simulink library "inp"  (Simulink 8.2)
settings: Solver=ode45  SolverMode=SingleTasking  StartTime=0.0  StopTime=10.0  RelTol=1e-3
undefined symbols (not defined in the file or in its folder): C_TICK_TIME_S, ENUM_FAULTSTATE_FAIL, ENUM_FAULTSTATE_INDETERMINATE, ENUM_FAULTSTATE_PASS, ENUM_KEYSWITCH_ON, FALSE, KINP_ABS1CANCommuOk_flg_ovrdflg, KINP_ABS1CANCommuOk_flg_ovrdval, KINP_ABS1CANPeriodTime_s, KINP_ABS1CANRolCntOk_flg_ovrdflg, KINP_ABS1CANRolCntOk_flg_ovrdval, KINP_ABS2CANChkSumOk_flg_ovrdflg, KINP_ABS2CANChkSumOk_flg_ovrdval, KINP_ABS2CANCommuOk_flg_ovrdflg, KINP_ABS2CANCommuOk_flg_ovrdval, KINP_ABS2CANPeriodTime_s, KINP_ABS2CANRolCntOk_flg_ovrdflg, KINP_ABS2CANRolCntOk_flg_ovrdval, KINP_ABS3CANCommuOk_flg_ovrdflg, KINP_ABS3CANCommuOk_flg_ovrdval, KINP_ABS4CANCommuOk_flg_ovrdflg, KINP_ABS4CANCommuOk_flg_ovrdval, KINP_ABSActive_flg_defval, KINP_ABSActive_flg_invalid, KINP_ABSActive_flg_ovrdflg, KINP_ABSActive_flg_ovrdval, KINP_ABSActive_flg_setvalid, KINP_ABSActive_flg_srvcflg, KINP_ABSActive_flg_srvcval, KINP_ABSCANAlive_flg_ovrdflg, KINP_ABSCANAlive_flg_ovrdval, KINP_ABSCANCommuOk_flg_ovrdflg, KINP_ABSCANCommuOk_flg_ovrdval, KINP_ABSCANValidOk_flg_ovrdflg, KINP_ABSCANValidOk_flg_ovrdval, KINP_ABSFault_flg_defval, KINP_ABSFault_flg_invalid, KINP_ABSFault_flg_ovrdflg, KINP_ABSFault_flg_ovrdval, KINP_ABSFault_flg_setvalid, KINP_ABSFault_flg_srvcflg, KINP_ABSFault_flg_srvcval, KINP_AC1CANCommuOk_flg_ovrdflg, KINP_AC1CANCommuOk_flg_ovrdval, KINP_ACCANAlive_flg_ovrdflg, KINP_ACCANAlive_flg_ovrdval, KINP_ACCANCommuOk_flg_ovrdflg, KINP_ACCANCommuOk_flg_ovrdval, KINP_ACCANValidOk_flg_ovrdflg, KINP_ACCANValidOk_flg_ovrdval, KINP_ACCCancelH_flg_defval, KINP_ACCCancelH_flg_invalid, KINP_ACCCancelH_flg_ovrdflg, KINP_ACCCancelH_flg_ovrdval, KINP_ACCCancelH_flg_setvalid, KINP_ACCCancelH_flg_srvcflg, KINP_ACCCancelH_flg_srvcval, KINP_AccelPedalPosn_pct_defval, KINP_AccelPedalPosn_pct_gain, KINP_AccelPedalPosn_pct_invalid ... (+1866)
libraries: inp x192, hcu_lib x34, simulink x33
(part 6 of 21)

== system inp/hw_signal_process/sig_valid_check ==
  input_signal_filt : Inport
  adc_fault : Inport  Port=2
  vref_fault : Inport  Port=3
  range_high_ifault_flg : Inport  Port=4
  range_low_ifault_flg : Inport  Port=5
  lim : Inport  Port=6
  inc : Inport  Port=7
  dec : Inport  Port=8
  def_value : Inport  Port=9
  Logical Operator1 : Logic  Operator=OR  Inputs=4  AllPortsSameDT=off  OutDataTypeStr=boolean
  Logical Operator2 : Logic  Operator=OR  Inputs=4  AllPortsSameDT=off  OutDataTypeStr=boolean
  Logical Operator3 : Logic  Operator=OR  AllPortsSameDT=off  OutDataTypeStr=boolean
  Relational Operator{,1..3} (4 alike) : RelationalOperator  Operator===  OutDataTypeStr=boolean
  Switch{,1..5} (6 alike) : Switch  Criteria=u2 ~= 0
  Terminator{1..4} (4 alike) : Terminator
  Unit Delay : UnitDelay  SampleTime=-1
  resetting_fault_filter{,1..3} (4 alike) : hcu_lib/fault_filter_reset
  zero{,1,4,8} (4 alike) : Constant  Tag=struct('comment',struct('text','','placement',1),'output',struct('width',1,'name','$S_$B','unit','','type','Int16','offset',0,'max',NaN,'min',NaN,'freeze',1,'lsb',1,'arb',0,'class','default','address','','description','','value','0','tolerance',1,'show',0),'date',731455.58675544)  Value=ENUM_FAULTSTATE_FAIL
  zero{2..3,6..7} (4 alike) : Constant  Tag=struct('comment',struct('text','','placement',1),'output',struct('width',1,'name','$S_$B','unit','','type','Int16','offset',0,'max',NaN,'min',NaN,'freeze',1,'lsb',1,'arb',0,'class','default','address','','description','','value','0','tolerance',1,'show',0),'date',731455.58675544)  Value=ENUM_FAULTSTATE_INDETERMINATE
  zero5 : Constant  Tag=struct('comment',struct('text','','placement',1),'output',struct('width',1,'name','$S_$B','unit','','type','Int16','offset',0,'max',NaN,'min',NaN,'freeze',1,'lsb',1,'arb',0,'class','default','address','','description','','value','0','tolerance',1,'show',0),'date',731455.58675544)  Value=ENUM_FAULTSTATE_PASS
  zero9 : Constant  Tag=struct('comment',struct('text','','placement',1),'output',struct('width',1,'name','$S_$B','unit','','type','Int16','offset',0,'max',NaN,'min',NaN,'freeze',1,'lsb',1,'arb',0,'class','default','address','','description','','value','0','tolerance',1,'show',0),'date',731455.58675544)  Value=ENUM_FAULTSTATE_PASS
  range_high_flt : Outport
  range_low_flt : Outport  Port=2
  fault_flg : Outport  Port=3
  valid_signal : Outport  Port=4
  wires:
  Unit Delay/1 -> Switch1/1
  [fault_flg] -> Switch/2
  [inst_low_fault] -> resetting_fault_filter2/1
  Switch/1 -> Unit Delay/1, valid_signal/1
  Switch1/1 -> Switch/3
  def_value/1 -> Switch/1
  dec/1 -> resetting_fault_filter/4, [dec]
  inc/1 -> resetting_fault_filter/3, [inc]
  lim/1 -> resetting_fault_filter/2, [lim]
  range_high_ifault_flg/1 -> [inst_high_fault]
  input_signal_filt/1 -> Switch1/3
  Logical Operator1/1 -> Switch1/2  "ifault_flg"
  range_low_ifault_flg/1 -> [inst_low_fault]
  resetting_fault_filter2/1 -> [low_fault_flg]
  resetting_fault_filter/2 -> Terminator1/1
  resetting_fault_filter2/2 -> Terminator2/1
  [lim] -> resetting_fault_filter2/2
  [inc] -> resetting_fault_filter2/3
  [dec] -> resetting_fault_filter2/4
  adc_fault/1 -> Relational Operator/1, [adc_fault]
  zero/1 -> Relational Operator/2
  zero1/1 -> Relational Operator1/2
  vref_fault/1 -> Relational Operator1/1, [vref_fault]
  Relational Operator/1 -> [inst_adc_fault]
  Relational Operator1/1 -> [inst_vref_fault]
  [lim] -> resetting_fault_filter1/2
  [inc] -> resetting_fault_filter1/3
  [dec] -> resetting_fault_filter1/4
  [inst_vref_fault] -> resetting_fault_filter3/1
  [lim] -> resetting_fault_filter3/2
  [inc] -> resetting_fault_filter3/3
  [dec] -> resetting_fault_filter3/4
  resetting_fault_filter1/2 -> Terminator3/1
  resetting_fault_filter3/2 -> Terminator4/1
  resetting_fault_filter3/1 -> [vref_fault_flg]
  [inst_adc_fault] -> resetting_fault_filter/1
  resetting_fault_filter/1 -> [adc_fault_flg]
  [inst_high_fault] -> resetting_fault_filter1/1
  resetting_fault_filter1/1 -> [high_fault_flg]
  [adc_fault_flg] -> Logical Operator2/1
  [vref_fault_flg] -> Logical Operator2/2
  [high_fault_flg] -> Logical Operator2/3
  [low_fault_flg] -> Logical Operator2/4
  Logical Operator2/1 -> fault_flg/1, [fault_flg]  "fault_flg"
  [inst_adc_fault] -> Logical Operator1/1
  [inst_vref_fault] -> Logical Operator1/2
  [inst_high_fault] -> Logical Operator1/3
  [inst_low_fault] -> Logical Operator1/4
  zero2/1 -> Relational Operator2/2
  [high_fault_flg] -> Switch3/2
  Switch3/1 -> Switch2/3
  zero3/1 -> Switch2/1
  [adc_fault] -> Relational Operator2/1
  zero4/1 -> Switch3/1
  zero5/1 -> Switch3/3
  Switch2/1 -> range_high_flt/1
  zero6/1 -> Relational Operator3/2
  [vref_fault] -> Relational Operator3/1
  Relational Operator2/1 -> Logical Operator3/1
  Relational Operator3/1 -> Logical Operator3/2
  [indeterminate] -> Switch2/2
  Logical Operator3/1 -> [indeterminate]
  [low_fault_flg] -> Switch5/2
  Switch5/1 -> Switch4/3
  zero7/1 -> Switch4/1
  zero8/1 -> Switch5/1
  zero9/1 -> Switch5/3
  [indeterminate] -> Switch4/2
  Switch4/1 -> range_low_flt/1

== system inp/hw_signal_process/signal_filter ==
  raw_input : Inport
  Tc : Inport  Port=2
  Ts : Inport  Port=3
  FirstOrderFilter : hcu_lib/FirstOrderFilter  SystemSampleTime=-1
  IC : UnitDelay  Tag=struct('comment',struct('text','','placement',1),'logdata',struct('maxnumvals',inf,'plotchannels',[],'stepsize',0,'downsampling',1,'loggingmode',3,'channels',1),'output',struct('width',1,'name','$S_$B','unit','','type','Int16','offset',0,'max',NaN,'min',NaN,'freeze',1,'lsb',1,'arb',0,'class','default','address','','description','','autoscalingmode',1,'lowerheadroom',1,'upperheadroom',1,'headroomunit','bit','checkmin',0,'checkmax',0,'show',0,'initial','2'),'state',0,'dt','-1','date',731455.586925799)  SampleTime=-1
  Switch1 : Switch  Tag=struct('comment',struct('text','','placement',1),'logdata',struct('maxnumvals',inf,'plotchannels',[],'stepsize',0,'downsampling',1,'loggingmode',3,'channels',1),'output',struct('width',1,'name','$S_$B','unit','','type','Int32Bin15','offset',0,'max',65535,'min',-65536,'freeze',1,'lsb',2^-15,'arb',0,'class','default','address','','description','','autoscalingmode',1,'lowerheadroom',1,'upperheadroom',1,'headroomunit','bit','checkmin',0,'checkmax',0,'show',0),'threshold',struct('width',1,'name','$S_$B_threshold','unit','','type','Int16','offset',0,'max',NaN,'min',NaN,'freeze',1,'lsb',1,'arb',0,'class','default','address','','description','','value','1','scalar',0),'date',731455.58657044)  Threshold=0.5  InputSameDT=on
  zero : Constant  Tag=struct('comment',struct('text','','placement',1),'output',struct('width',1,'name','$S_$B','unit','','type','Int16','offset',0,'max',NaN,'min',NaN,'freeze',1,'lsb',1,'arb',0,'class','default','address','','description','','value','0','tolerance',1,'show',0),'date',731455.58675544)  Value=TRUE
  raw_input_filt : Outport
  wires:
  Switch1/1 -> raw_input_filt/1
  FirstOrderFilter/1 -> Switch1/1
  IC/1 -> Switch1/2
  Ts/1 -> FirstOrderFilter/3
  Tc/1 -> FirstOrderFilter/2
  raw_input/1 -> Switch1/3, FirstOrderFilter/1
  zero/1 -> IC/1

== system inp/INP/ABS_signals/ABSActive ==
  signal_in : Inport
  msg_valid : Inport  Port=2
  Active_fault_flg : Inport  Port=3
  can_signal_process_wio_validity : inp/can_signal_process_wio_validity
  defval : Constant  Value=KINP_ABSActive_flg_defval
  invalid : Constant  Value=KINP_ABSActive_flg_invalid
  ovrdflg : Constant  Value=KINP_ABSActive_flg_ovrdflg
  ovrdval : Constant  Value=KINP_ABSActive_flg_ovrdval
  setvalid : Constant  Value=KINP_ABSActive_flg_setvalid
  srvcflg : Constant  Value=KINP_ABSActive_flg_srvcflg
  srvcval : Constant  Value=KINP_ABSActive_flg_srvcval
  signal_out : Outport
  signal_valid_out : Outport  Port=2
  wires:
  Active_fault_flg/1 -> can_signal_process_wio_validity/3
  srvcval/1 -> can_signal_process_wio_validity/10
  srvcflg/1 -> can_signal_process_wio_validity/9
  ovrdval/1 -> can_signal_process_wio_validity/8
  ovrdflg/1 -> can_signal_process_wio_validity/7
  setvalid/1 -> can_signal_process_wio_validity/6
  invalid/1 -> can_signal_process_wio_validity/5
  defval/1 -> can_signal_process_wio_validity/4
  msg_valid/1 -> can_signal_process_wio_validity/2
  signal_in/1 -> can_signal_process_wio_validity/1
  can_signal_process_wio_validity/2 -> signal_valid_out/1
  can_signal_process_wio_validity/1 -> signal_out/1

== system inp/INP/ABS_signals/ABSFault ==
  signal_in : Inport
  msg_valid : Inport  Port=2
  Active_fault_flg : Inport  Port=3
  can_signal_process_wio_validity : inp/can_signal_process_wio_validity
  defval : Constant  Value=KINP_ABSFault_flg_defval
  invalid : Constant  Value=KINP_ABSFault_flg_invalid
  ovrdflg : Constant  Value=KINP_ABSFault_flg_ovrdflg
  ovrdval : Constant  Value=KINP_ABSFault_flg_ovrdval
  setvalid : Constant  Value=KINP_ABSFault_flg_setvalid
  srvcflg : Constant  Value=KINP_ABSFault_flg_srvcflg
  srvcval : Constant  Value=KINP_ABSFault_flg_srvcval
  signal_out : Outport
  signal_valid_out : Outport  Port=2
  wires:
  can_signal_process_wio_validity/1 -> signal_out/1
  can_signal_process_wio_validity/2 -> signal_valid_out/1
  signal_in/1 -> can_signal_process_wio_validity/1
  msg_valid/1 -> can_signal_process_wio_validity/2
  defval/1 -> can_signal_process_wio_validity/4
  invalid/1 -> can_signal_process_wio_validity/5
  setvalid/1 -> can_signal_process_wio_validity/6
  ovrdflg/1 -> can_signal_process_wio_validity/7
  ovrdval/1 -> can_signal_process_wio_validity/8
  srvcflg/1 -> can_signal_process_wio_validity/9
  srvcval/1 -> can_signal_process_wio_validity/10
  Active_fault_flg/1 -> can_signal_process_wio_validity/3

== system inp/INP/ABS_signals/BrakBoosterPres ==
  signal_in : Inport
  signal_valid_in : Inport  Port=2
  msg_valid : Inport  Port=3
  Active_fault_flg : Inport  Port=4
  can_signal_process_with_validity : inp/can_signal_process_with_validity
  conv_gain_offset : Subsystem
  defval : Constant  Value=KINP_BrakBoosterPres_kPa_defval
  invalid : Constant  Value=KINP_BrakBoosterPres_kPa_invalid
  min_max_limit : Subsystem
  ovrdflg : Constant  Value=KINP_BrakBoosterPres_kPa_ovrdflg
  ovrdval : Constant  Value=KINP_BrakBoosterPres_kPa_ovrdval
  setvalid : Constant  Value=KINP_BrakBoosterPres_kPa_setvalid
  srvcflg : Constant  Value=KINP_BrakBoosterPres_kPa_srvcflg
  srvcval : Constant  Value=KINP_BrakBoosterPres_kPa_srvcval
  signal_out : Outport
  signal_valid_out : Outport  Port=2
  raw_signal_valid_out : Outport  Port=3
  wires:
  can_signal_process_with_validity/3 -> raw_signal_valid_out/1
  can_signal_process_with_validity/1 -> min_max_limit/1(in)
  can_signal_process_with_validity/2 -> signal_valid_out/1
  signal_in/1 -> conv_gain_offset/1(in)
  signal_valid_in/1 -> can_signal_process_with_validity/2
  msg_valid/1 -> can_signal_process_with_validity/5, can_signal_process_with_validity/3
  defval/1 -> can_signal_process_with_validity/6
  invalid/1 -> can_signal_process_with_validity/7
  conv_gain_offset/1(out) -> can_signal_process_with_validity/1
  min_max_limit/1(out) -> signal_out/1
  setvalid/1 -> can_signal_process_with_validity/8
  ovrdflg/1 -> can_signal_process_with_validity/9
  ovrdval/1 -> can_signal_process_with_validity/10
  srvcflg/1 -> can_signal_process_with_validity/11
  srvcval/1 -> can_signal_process_with_validity/12
  Active_fault_flg/1 -> can_signal_process_with_validity/4

== system inp/INP/ABS_signals/BrkLampOn ==
  signal_in : Inport
  signal_valid_in : Inport  Port=2
  msg_valid : Inport  Port=3
  Active_fault_flg : Inport  Port=4
  can_signal_process_with_validity : inp/can_signal_process_with_validity
  defval : Constant  Value=KINP_BrkLampOn_flg_defval
  invalid : Constant  Value=KINP_BrkLampOn_flg_invalid
  ovrdflg : Constant  Value=KINP_BrkLampOn_flg_ovrdflg
  ovrdval : Constant  Value=KINP_BrkLampOn_flg_ovrdval
  setvalid : Constant  Value=KINP_BrkLampOn_flg_setvalid
  srvcflg : Constant  Value=KINP_BrkLampOn_flg_srvcflg
  srvcval : Constant  Value=KINP_BrkLampOn_flg_srvcval
  signal_out : Outport
  signal_valid_out : Outport  Port=2
  raw_signal_valid_out : Outport  Port=3
  wires:
  Active_fault_flg/1 -> can_signal_process_with_validity/4
  srvcval/1 -> can_signal_process_with_validity/12
  srvcflg/1 -> can_signal_process_with_validity/11
  ovrdval/1 -> can_signal_process_with_validity/10
  ovrdflg/1 -> can_signal_process_with_validity/9
  setvalid/1 -> can_signal_process_with_validity/8
  invalid/1 -> can_signal_process_with_validity/7
  defval/1 -> can_signal_process_with_validity/6
  msg_valid/1 -> can_signal_process_with_validity/3, can_signal_process_with_validity/5
  signal_valid_in/1 -> can_signal_process_with_validity/2
  signal_in/1 -> can_signal_process_with_validity/1
  can_signal_process_with_validity/2 -> signal_valid_out/1
  can_signal_process_with_validity/1 -> signal_out/1
  can_signal_process_with_validity/3 -> raw_signal_valid_out/1

== system inp/INP/ABS_signals/EBDFault ==
  signal_in : Inport
  msg_valid : Inport  Port=2
  Active_fault_flg : Inport  Port=3
  can_signal_process_wio_validity : inp/can_signal_process_wio_validity
  defval : Constant  Value=KINP_EBDFault_flg_defval
  invalid : Constant  Value=KINP_EBDFault_flg_invalid
  ovrdflg : Constant  Value=KINP_EBDFault_flg_ovrdflg
  ovrdval : Constant  Value=KINP_EBDFault_flg_ovrdval
  setvalid : Constant  Value=KINP_EBDFault_flg_setvalid
  srvcflg : Constant  Value=KINP_EBDFault_flg_srvcflg
  srvcval : Constant  Value=KINP_EBDFault_flg_srvcval
  signal_out : Outport
  signal_valid_out : Outport  Port=2
  wires:
  can_signal_process_wio_validity/1 -> signal_out/1
  can_signal_process_wio_validity/2 -> signal_valid_out/1
  signal_in/1 -> can_signal_process_wio_validity/1
  msg_valid/1 -> can_signal_process_wio_validity/2
  defval/1 -> can_signal_process_wio_validity/4
  invalid/1 -> can_signal_process_wio_validity/5
  setvalid/1 -> can_signal_process_wio_validity/6
  ovrdflg/1 -> can_signal_process_wio_validity/7
  ovrdval/1 -> can_signal_process_wio_validity/8
  srvcflg/1 -> can_signal_process_wio_validity/9
  srvcval/1 -> can_signal_process_wio_validity/10
  Active_fault_flg/1 -> can_signal_process_wio_validity/3

== system inp/INP/ABS_signals/ESCActive ==
  signal_in : Inport
  msg_valid : Inport  Port=2
  Active_fault_flg : Inport  Port=3
  can_signal_process_wio_validity : inp/can_signal_process_wio_validity
  defval : Constant  Value=KINP_ESCActive_flg_defval
  invalid : Constant  Value=KINP_ESCActive_flg_invalid
  ovrdflg : Constant  Value=KINP_ESCActive_flg_ovrdflg
  ovrdval : Constant  Value=KINP_ESCActive_flg_ovrdval
  setvalid : Constant  Value=KINP_ESCActive_flg_setvalid
  srvcflg : Constant  Value=KINP_ESCActive_flg_srvcflg
  srvcval : Constant  Value=KINP_ESCActive_flg_srvcval
  signal_out : Outport
  signal_valid_out : Outport  Port=2
  wires:
  Active_fault_flg/1 -> can_signal_process_wio_validity/3
  srvcval/1 -> can_signal_process_wio_validity/10
  srvcflg/1 -> can_signal_process_wio_validity/9
  ovrdval/1 -> can_signal_process_wio_validity/8
  ovrdflg/1 -> can_signal_process_wio_validity/7
  setvalid/1 -> can_signal_process_wio_validity/6
  invalid/1 -> can_signal_process_wio_validity/5
  defval/1 -> can_signal_process_wio_validity/4
  msg_valid/1 -> can_signal_process_wio_validity/2
  signal_in/1 -> can_signal_process_wio_validity/1
  can_signal_process_wio_validity/2 -> signal_valid_out/1
  can_signal_process_wio_validity/1 -> signal_out/1

== system inp/INP/ABS_signals/ESCFault ==
  signal_in : Inport
  msg_valid : Inport  Port=2
  Active_fault_flg : Inport  Port=3
  can_signal_process_wio_validity : inp/can_signal_process_wio_validity
  defval : Constant  Value=KINP_ESCFault_flg_defval
  invalid : Constant  Value=KINP_ESCFault_flg_invalid
  ovrdflg : Constant  Value=KINP_ESCFault_flg_ovrdflg
  ovrdval : Constant  Value=KINP_ESCFault_flg_ovrdval
  setvalid : Constant  Value=KINP_ESCFault_flg_setvalid
  srvcflg : Constant  Value=KINP_ESCFault_flg_srvcflg
  srvcval : Constant  Value=KINP_ESCFault_flg_srvcval
  signal_out : Outport
  signal_valid_out : Outport  Port=2
  wires:
  can_signal_process_wio_validity/1 -> signal_out/1
  can_signal_process_wio_validity/2 -> signal_valid_out/1
  signal_in/1 -> can_signal_process_wio_validity/1
  msg_valid/1 -> can_signal_process_wio_validity/2
  defval/1 -> can_signal_process_wio_validity/4
  invalid/1 -> can_signal_process_wio_validity/5
  setvalid/1 -> can_signal_process_wio_validity/6
  ovrdflg/1 -> can_signal_process_wio_validity/7
  ovrdval/1 -> can_signal_process_wio_validity/8
  srvcflg/1 -> can_signal_process_wio_validity/9
  srvcval/1 -> can_signal_process_wio_validity/10
  Active_fault_flg/1 -> can_signal_process_wio_validity/3

== system inp/INP/ABS_signals/HSAActive ==
  signal_in : Inport
  msg_valid : Inport  Port=2
  Active_fault_flg : Inport  Port=3
  can_signal_process_wio_validity : inp/can_signal_process_wio_validity
  defval : Constant  Value=KINP_HSAActive_flg_defval
  invalid : Constant  Value=KINP_HSAActive_flg_invalid
  ovrdflg : Constant  Value=KINP_HSAActive_flg_ovrdflg
  ovrdval : Constant  Value=KINP_HSAActive_flg_ovrdval
  setvalid : Constant  Value=KINP_HSAActive_flg_setvalid
  srvcflg : Constant  Value=KINP_HSAActive_flg_srvcflg
  srvcval : Constant  Value=KINP_HSAActive_flg_srvcval
  signal_out : Outport
  signal_valid_out : Outport  Port=2
  wires:
  can_signal_process_wio_validity/1 -> signal_out/1
  can_signal_process_wio_validity/2 -> signal_valid_out/1
  signal_in/1 -> can_signal_process_wio_validity/1
  msg_valid/1 -> can_signal_process_wio_validity/2
  defval/1 -> can_signal_process_wio_validity/4
  invalid/1 -> can_signal_process_wio_validity/5
  setvalid/1 -> can_signal_process_wio_validity/6
  ovrdflg/1 -> can_signal_process_wio_validity/7
  ovrdval/1 -> can_signal_process_wio_validity/8
  srvcflg/1 -> can_signal_process_wio_validity/9
  srvcval/1 -> can_signal_process_wio_validity/10
  Active_fault_flg/1 -> can_signal_process_wio_validity/3

== system inp/INP/ABS_signals/HSAFault ==
  signal_in : Inport
  msg_valid : Inport  Port=2
  Active_fault_flg : Inport  Port=3
  can_signal_process_wio_validity : inp/can_signal_process_wio_validity
  defval : Constant  Value=KINP_HSAFault_flg_defval
  invalid : Constant  Value=KINP_HSAFault_flg_invalid
  ovrdflg : Constant  Value=KINP_HSAFault_flg_ovrdflg
  ovrdval : Constant  Value=KINP_HSAFault_flg_ovrdval
  setvalid : Constant  Value=KINP_HSAFault_flg_setvalid
  srvcflg : Constant  Value=KINP_HSAFault_flg_srvcflg
  srvcval : Constant  Value=KINP_HSAFault_flg_srvcval
  signal_out : Outport
  signal_valid_out : Outport  Port=2
  wires:
  Active_fault_flg/1 -> can_signal_process_wio_validity/3
  srvcval/1 -> can_signal_process_wio_validity/10
  srvcflg/1 -> can_signal_process_wio_validity/9
  ovrdval/1 -> can_signal_process_wio_validity/8
  ovrdflg/1 -> can_signal_process_wio_validity/7
  setvalid/1 -> can_signal_process_wio_validity/6
  invalid/1 -> can_signal_process_wio_validity/5
  defval/1 -> can_signal_process_wio_validity/4
  msg_valid/1 -> can_signal_process_wio_validity/2
  signal_in/1 -> can_signal_process_wio_validity/1
  can_signal_process_wio_validity/2 -> signal_valid_out/1
  can_signal_process_wio_validity/1 -> signal_out/1

== system inp/INP/ABS_signals/LFWheelSpeed ==
  signal_in : Inport
  signal_valid_in : Inport  Port=2
  msg_valid : Inport  Port=3
  Active_fault_flg : Inport  Port=4
  can_signal_process_with_validity : inp/can_signal_process_with_validity
  conv_gain_offset : Subsystem
  defval : Constant  Value=KINP_LFWheelSpeed_kph_defval
  invalid : Constant  Value=KINP_LFWheelSpeed_kph_invalid
  min_max_limit : Subsystem
  ovrdflg : Constant  Value=KINP_LFWheelSpeed_kph_ovrdflg
  ovrdval : Constant  Value=KINP_LFWheelSpeed_kph_ovrdval
  setvalid : Constant  Value=KINP_LFWheelSpeed_kph_setvalid
  srvcflg : Constant  Value=KINP_LFWheelSpeed_kph_srvcflg
  srvcval : Constant  Value=KINP_LFWheelSpeed_kph_srvcval
  signal_out : Outport
  signal_valid_out : Outport  Port=2
  raw_signal_valid_out : Outport  Port=3
  wires:
  can_signal_process_with_validity/3 -> raw_signal_valid_out/1
  can_signal_process_with_validity/1 -> min_max_limit/1(in)
  can_signal_process_with_validity/2 -> signal_valid_out/1
  signal_in/1 -> conv_gain_offset/1(in)
  signal_valid_in/1 -> can_signal_process_with_validity/2
  msg_valid/1 -> can_signal_process_with_validity/5, can_signal_process_with_validity/3
  defval/1 -> can_signal_process_with_validity/6
  invalid/1 -> can_signal_process_with_validity/7
  conv_gain_offset/1(out) -> can_signal_process_with_validity/1
  min_max_limit/1(out) -> signal_out/1
  setvalid/1 -> can_signal_process_with_validity/8
  ovrdflg/1 -> can_signal_process_with_validity/9
  ovrdval/1 -> can_signal_process_with_validity/10
  srvcflg/1 -> can_signal_process_with_validity/11
  srvcval/1 -> can_signal_process_with_validity/12
  Active_fault_flg/1 -> can_signal_process_with_validity/4

== system inp/INP/ABS_signals/LRWheelSpeed ==
  signal_in : Inport
  signal_valid_in : Inport  Port=2
  msg_valid : Inport  Port=3
  Active_fault_flg : Inport  Port=4
  can_signal_process_with_validity : inp/can_signal_process_with_validity
  conv_gain_offset : Subsystem
  defval : Constant  Value=KINP_LRWheelSpeed_kph_defval
  invalid : Constant  Value=KINP_LRWheelSpeed_kph_invalid
  min_max_limit : Subsystem
  ovrdflg : Constant  Value=KINP_LRWheelSpeed_kph_ovrdflg
  ovrdval : Constant  Value=KINP_LRWheelSpeed_kph_ovrdval
  setvalid : Constant  Value=KINP_LRWheelSpeed_kph_setvalid
  srvcflg : Constant  Value=KINP_LRWheelSpeed_kph_srvcflg
  srvcval : Constant  Value=KINP_LRWheelSpeed_kph_srvcval
  signal_out : Outport
  signal_valid_out : Outport  Port=2
  raw_signal_valid_out : Outport  Port=3
  wires:
  Active_fault_flg/1 -> can_signal_process_with_validity/4
  srvcval/1 -> can_signal_process_with_validity/12
  srvcflg/1 -> can_signal_process_with_validity/11
  ovrdval/1 -> can_signal_process_with_validity/10
  ovrdflg/1 -> can_signal_process_with_validity/9
  setvalid/1 -> can_signal_process_with_validity/8
  min_max_limit/1(out) -> signal_out/1
  conv_gain_offset/1(out) -> can_signal_process_with_validity/1
  invalid/1 -> can_signal_process_with_validity/7
  defval/1 -> can_signal_process_with_validity/6
  msg_valid/1 -> can_signal_process_with_validity/3, can_signal_process_with_validity/5
  signal_valid_in/1 -> can_signal_process_with_validity/2
  signal_in/1 -> conv_gain_offset/1(in)
  can_signal_process_with_validity/2 -> signal_valid_out/1
  can_signal_process_with_validity/1 -> min_max_limit/1(in)
  can_signal_process_with_validity/3 -> raw_signal_valid_out/1

== system inp/INP/ABS_signals/LatAccel ==
  signal_in : Inport
  signal_valid_in : Inport  Port=2
  msg_valid : Inport  Port=3
  Active_fault_flg : Inport  Port=4
  can_signal_process_with_validity : inp/can_signal_process_with_validity
  conv_gain_offset : Subsystem
  defval : Constant  Value=KINP_LatAccel_G_defval
  invalid : Constant  Value=KINP_LatAccel_G_invalid
  min_max_limit : Subsystem
  ovrdflg : Constant  Value=KINP_LatAccel_G_ovrdflg
  ovrdval : Constant  Value=KINP_LatAccel_G_ovrdval
  setvalid : Constant  Value=KINP_LatAccel_G_setvalid
  srvcflg : Constant  Value=KINP_LatAccel_G_srvcflg
  srvcval : Constant  Value=KINP_LatAccel_G_srvcval
  signal_out : Outport
  signal_valid_out : Outport  Port=2
  raw_signal_valid_out : Outport  Port=3
  wires:
  can_signal_process_with_validity/3 -> raw_signal_valid_out/1
  can_signal_process_with_validity/1 -> min_max_limit/1(in)
  can_signal_process_with_validity/2 -> signal_valid_out/1
  signal_in/1 -> conv_gain_offset/1(in)
  signal_valid_in/1 -> can_signal_process_with_validity/2
  msg_valid/1 -> can_signal_process_with_validity/5, can_signal_process_with_validity/3
  defval/1 -> can_signal_process_with_validity/6
  invalid/1 -> can_signal_process_with_validity/7
  conv_gain_offset/1(out) -> can_signal_process_with_validity/1
  min_max_limit/1(out) -> signal_out/1
  setvalid/1 -> can_signal_process_with_validity/8
  ovrdflg/1 -> can_signal_process_with_validity/9
  ovrdval/1 -> can_signal_process_with_validity/10
  srvcflg/1 -> can_signal_process_with_validity/11
  srvcval/1 -> can_signal_process_with_validity/12
  Active_fault_flg/1 -> can_signal_process_with_validity/4

== system inp/INP/ABS_signals/LongAccel ==
  signal_in : Inport
  signal_valid_in : Inport  Port=2
  msg_valid : Inport  Port=3
  Active_fault_flg : Inport  Port=4
  can_signal_process_with_validity : inp/can_signal_process_with_validity
  conv_gain_offset : Subsystem
  defval : Constant  Value=KINP_LongAccel_G_defval
  invalid : Constant  Value=KINP_LongAccel_G_invalid
  min_max_limit : Subsystem
  ovrdflg : Constant  Value=KINP_LongAccel_G_ovrdflg
  ovrdval : Constant  Value=KINP_LongAccel_G_ovrdval
  setvalid : Constant  Value=KINP_LongAccel_G_setvalid
  srvcflg : Constant  Value=KINP_LongAccel_G_srvcflg
  srvcval : Constant  Value=KINP_LongAccel_G_srvcval
  signal_out : Outport
  signal_valid_out : Outport  Port=2
  raw_signal_valid_out : Outport  Port=3
  wires:
  Active_fault_flg/1 -> can_signal_process_with_validity/4
  srvcval/1 -> can_signal_process_with_validity/12
  srvcflg/1 -> can_signal_process_with_validity/11
  ovrdval/1 -> can_signal_process_with_validity/10
  ovrdflg/1 -> can_signal_process_with_validity/9
  setvalid/1 -> can_signal_process_with_validity/8
  min_max_limit/1(out) -> signal_out/1
  conv_gain_offset/1(out) -> can_signal_process_with_validity/1
  invalid/1 -> can_signal_process_with_validity/7
  defval/1 -> can_signal_process_with_validity/6
  msg_valid/1 -> can_signal_process_with_validity/3, can_signal_process_with_validity/5
  signal_valid_in/1 -> can_signal_process_with_validity/2
  signal_in/1 -> conv_gain_offset/1(in)
  can_signal_process_with_validity/2 -> signal_valid_out/1
  can_signal_process_with_validity/1 -> min_max_limit/1(in)
  can_signal_process_with_validity/3 -> raw_signal_valid_out/1

== system inp/INP/ABS_signals/LowBrakBoosterPres ==
  signal_in : Inport
  msg_valid : Inport  Port=2
  Active_fault_flg : Inport  Port=3
  can_signal_process_wio_validity : inp/can_signal_process_wio_validity
  defval : Constant  Value=KINP_LowBrakBoosterPres_flg_defval
  invalid : Constant  Value=KINP_LowBrakBoosterPres_flg_invalid
  ovrdflg : Constant  Value=KINP_LowBrakBoosterPres_flg_ovrdflg
  ovrdval : Constant  Value=KINP_LowBrakBoosterPres_flg_ovrdval
  setvalid : Constant  Value=KINP_LowBrakBoosterPres_flg_setvalid
  srvcflg : Constant  Value=KINP_LowBrakBoosterPres_flg_srvcflg
  srvcval : Constant  Value=KINP_LowBrakBoosterPres_flg_srvcval
  signal_out : Outport
  signal_valid_out : Outport  Port=2
  wires:
  Active_fault_flg/1 -> can_signal_process_wio_validity/3
  srvcval/1 -> can_signal_process_wio_validity/10
  srvcflg/1 -> can_signal_process_wio_validity/9
  ovrdval/1 -> can_signal_process_wio_validity/8
  ovrdflg/1 -> can_signal_process_wio_validity/7
  setvalid/1 -> can_signal_process_wio_validity/6
  invalid/1 -> can_signal_process_wio_validity/5
  defval/1 -> can_signal_process_wio_validity/4
  msg_valid/1 -> can_signal_process_wio_validity/2
  signal_in/1 -> can_signal_process_wio_validity/1
  can_signal_process_wio_validity/2 -> signal_valid_out/1
  can_signal_process_wio_validity/1 -> signal_out/1

== system inp/INP/ABS_signals/MCPressure ==
  signal_in : Inport
  signal_valid_in : Inport  Port=2
  msg_valid : Inport  Port=3
  Active_fault_flg : Inport  Port=4
  can_signal_process_with_validity : inp/can_signal_process_with_validity
  conv_gain_offset : Subsystem
  defval : Constant  Value=KINP_MCPressure_bar_defval
  invalid : Constant  Value=KINP_MCPressure_bar_invalid
  min_max_limit : Subsystem
  ovrdflg : Constant  Value=KINP_MCPressure_bar_ovrdflg
  ovrdval : Constant  Value=KINP_MCPressure_bar_ovrdval
  setvalid : Constant  Value=KINP_MCPressure_bar_setvalid
  srvcflg : Constant  Value=KINP_MCPressure_bar_srvcflg
  srvcval : Constant  Value=KINP_MCPressure_bar_srvcval
  signal_out : Outport
  signal_valid_out : Outport  Port=2
  raw_signal_valid_out : Outport  Port=3
  wires:
  can_signal_process_with_validity/3 -> raw_signal_valid_out/1
  can_signal_process_with_validity/1 -> min_max_limit/1(in)
  can_signal_process_with_validity/2 -> signal_valid_out/1
  signal_in/1 -> conv_gain_offset/1(in)
  signal_valid_in/1 -> can_signal_process_with_validity/2
  msg_valid/1 -> can_signal_process_with_validity/5, can_signal_process_with_validity/3
  defval/1 -> can_signal_process_with_validity/6
  invalid/1 -> can_signal_process_with_validity/7
  conv_gain_offset/1(out) -> can_signal_process_with_validity/1
  min_max_limit/1(out) -> signal_out/1
  setvalid/1 -> can_signal_process_with_validity/8
  ovrdflg/1 -> can_signal_process_with_validity/9
  ovrdval/1 -> can_signal_process_with_validity/10
  srvcflg/1 -> can_signal_process_with_validity/11
  srvcval/1 -> can_signal_process_with_validity/12
  Active_fault_flg/1 -> can_signal_process_with_validity/4

== system inp/INP/ABS_signals/RFWheelSpeed ==
  signal_in : Inport
  signal_valid_in : Inport  Port=2
  msg_valid : Inport  Port=3
  Active_fault_flg : Inport  Port=4
  can_signal_process_with_validity : inp/can_signal_process_with_validity
  conv_gain_offset : Subsystem
  defval : Constant  Value=KINP_RFWheelSpeed_kph_defval
  invalid : Constant  Value=KINP_RFWheelSpeed_kph_invalid
  min_max_limit : Subsystem
  ovrdflg : Constant  Value=KINP_RFWheelSpeed_kph_ovrdflg
  ovrdval : Constant  Value=KINP_RFWheelSpeed_kph_ovrdval
  setvalid : Constant  Value=KINP_RFWheelSpeed_kph_setvalid
  srvcflg : Constant  Value=KINP_RFWheelSpeed_kph_srvcflg
  srvcval : Constant  Value=KINP_RFWheelSpeed_kph_srvcval
  signal_out : Outport
  signal_valid_out : Outport  Port=2
  raw_signal_valid_out : Outport  Port=3
  wires:
  can_signal_process_with_validity/3 -> raw_signal_valid_out/1
  can_signal_process_with_validity/1 -> min_max_limit/1(in)
  can_signal_process_with_validity/2 -> signal_valid_out/1
  signal_in/1 -> conv_gain_offset/1(in)
  signal_valid_in/1 -> can_signal_process_with_validity/2
  msg_valid/1 -> can_signal_process_with_validity/5, can_signal_process_with_validity/3
  defval/1 -> can_signal_process_with_validity/6
  invalid/1 -> can_signal_process_with_validity/7
  conv_gain_offset/1(out) -> can_signal_process_with_validity/1
  min_max_limit/1(out) -> signal_out/1
  setvalid/1 -> can_signal_process_with_validity/8
  ovrdflg/1 -> can_signal_process_with_validity/9
  ovrdval/1 -> can_signal_process_with_validity/10
  srvcflg/1 -> can_signal_process_with_validity/11
  srvcval/1 -> can_signal_process_with_validity/12
  Active_fault_flg/1 -> can_signal_process_with_validity/4

== system inp/INP/ABS_signals/RRWheelSpeed ==
  signal_in : Inport
  signal_valid_in : Inport  Port=2
  msg_valid : Inport  Port=3
  Active_fault_flg : Inport  Port=4
  can_signal_process_with_validity : inp/can_signal_process_with_validity
  conv_gain_offset : Subsystem
  defval : Constant  Value=KINP_RRWheelSpeed_kph_defval
  invalid : Constant  Value=KINP_RRWheelSpeed_kph_invalid
  min_max_limit : Subsystem
  ovrdflg : Constant  Value=KINP_RRWheelSpeed_kph_ovrdflg
  ovrdval : Constant  Value=KINP_RRWheelSpeed_kph_ovrdval
  setvalid : Constant  Value=KINP_RRWheelSpeed_kph_setvalid
  srvcflg : Constant  Value=KINP_RRWheelSpeed_kph_srvcflg
  srvcval : Constant  Value=KINP_RRWheelSpeed_kph_srvcval
  signal_out : Outport
  signal_valid_out : Outport  Port=2
  raw_signal_valid_out : Outport  Port=3
  wires:
  Active_fault_flg/1 -> can_signal_process_with_validity/4
  srvcval/1 -> can_signal_process_with_validity/12
  srvcflg/1 -> can_signal_process_with_validity/11
  ovrdval/1 -> can_signal_process_with_validity/10
  ovrdflg/1 -> can_signal_process_with_validity/9
  setvalid/1 -> can_signal_process_with_validity/8
  min_max_limit/1(out) -> signal_out/1
  conv_gain_offset/1(out) -> can_signal_process_with_validity/1
  invalid/1 -> can_signal_process_with_validity/7
  defval/1 -> can_signal_process_with_validity/6
  msg_valid/1 -> can_signal_process_with_validity/3, can_signal_process_with_validity/5
  signal_valid_in/1 -> can_signal_process_with_validity/2
  signal_in/1 -> conv_gain_offset/1(in)
  can_signal_process_with_validity/2 -> signal_valid_out/1
  can_signal_process_with_validity/1 -> min_max_limit/1(in)
  can_signal_process_with_validity/3 -> raw_signal_valid_out/1

== system inp/INP/ABS_signals/SurfaceGrade ==
  signal_in : Inport
  signal_valid_in : Inport  Port=2
  msg_valid : Inport  Port=3
  Active_fault_flg : Inport  Port=4
  can_signal_process_with_validity : inp/can_signal_process_with_validity
  conv_gain_offset : Subsystem
  defval : Constant  Value=KINP_SurfaceGrade_deg_defval
  invalid : Constant  Value=KINP_SurfaceGrade_deg_invalid
  min_max_limit : Subsystem
  ovrdflg : Constant  Value=KINP_SurfaceGrade_deg_ovrdflg
  ovrdval : Constant  Value=KINP_SurfaceGrade_deg_ovrdval
  setvalid : Constant  Value=KINP_SurfaceGrade_deg_setvalid
  srvcflg : Constant  Value=KINP_SurfaceGrade_deg_srvcflg
  srvcval : Constant  Value=KINP_SurfaceGrade_deg_srvcval
  signal_out : Outport
  signal_valid_out : Outport  Port=2
  raw_signal_valid_out : Outport  Port=3
  wires:
  Active_fault_flg/1 -> can_signal_process_with_validity/4
  srvcval/1 -> can_signal_process_with_validity/12
  srvcflg/1 -> can_signal_process_with_validity/11
  ovrdval/1 -> can_signal_process_with_validity/10
  ovrdflg/1 -> can_signal_process_with_validity/9
  setvalid/1 -> can_signal_process_with_validity/8
  min_max_limit/1(out) -> signal_out/1
  conv_gain_offset/1(out) -> can_signal_process_with_validity/1
  invalid/1 -> can_signal_process_with_validity/7
  defval/1 -> can_signal_process_with_validity/6
  msg_valid/1 -> can_signal_process_with_validity/3, can_signal_process_with_validity/5
  signal_valid_in/1 -> can_signal_process_with_validity/2
  signal_in/1 -> conv_gain_offset/1(in)
  can_signal_process_with_validity/2 -> signal_valid_out/1
  can_signal_process_with_validity/1 -> min_max_limit/1(in)
  can_signal_process_with_validity/3 -> raw_signal_valid_out/1

== system inp/INP/ABS_signals/TCSActive ==
  signal_in : Inport
  msg_valid : Inport  Port=2
  Active_fault_flg : Inport  Port=3
  can_signal_process_wio_validity : inp/can_signal_process_wio_validity
  defval : Constant  Value=KINP_TCSActive_flg_defval
  invalid : Constant  Value=KINP_TCSActive_flg_invalid
  ovrdflg : Constant  Value=KINP_TCSActive_flg_ovrdflg
  ovrdval : Constant  Value=KINP_TCSActive_flg_ovrdval
  setvalid : Constant  Value=KINP_TCSActive_flg_setvalid
  srvcflg : Constant  Value=KINP_TCSActive_flg_srvcflg
  srvcval : Constant  Value=KINP_TCSActive_flg_srvcval
  signal_out : Outport
  signal_valid_out : Outport  Port=2
  wires:
  Active_fault_flg/1 -> can_signal_process_wio_validity/3
  srvcval/1 -> can_signal_process_wio_validity/10
  srvcflg/1 -> can_signal_process_wio_validity/9
  ovrdval/1 -> can_signal_process_wio_validity/8
  ovrdflg/1 -> can_signal_process_wio_validity/7
  setvalid/1 -> can_signal_process_wio_validity/6
  invalid/1 -> can_signal_process_wio_validity/5
  defval/1 -> can_signal_process_wio_validity/4
  msg_valid/1 -> can_signal_process_wio_validity/2
  signal_in/1 -> can_signal_process_wio_validity/1
  can_signal_process_wio_validity/2 -> signal_valid_out/1
  can_signal_process_wio_validity/1 -> signal_out/1

== system inp/INP/ABS_signals/TCSEnabled ==
  signal_in : Inport
  msg_valid : Inport  Port=2
  Active_fault_flg : Inport  Port=3
  can_signal_process_wio_validity : inp/can_signal_process_wio_validity
  defval : Constant  Value=KINP_TCSEnabled_flg_defval
  invalid : Constant  Value=KINP_TCSEnabled_flg_invalid
  ovrdflg : Constant  Value=KINP_TCSEnabled_flg_ovrdflg
  ovrdval : Constant  Value=KINP_TCSEnabled_flg_ovrdval
  setvalid : Constant  Value=KINP_TCSEnabled_flg_setvalid
  srvcflg : Constant  Value=KINP_TCSEnabled_flg_srvcflg
  srvcval : Constant  Value=KINP_TCSEnabled_flg_srvcval
  signal_out : Outport
  signal_valid_out : Outport  Port=2
  wires:
  can_signal_process_wio_validity/1 -> signal_out/1
  can_signal_process_wio_validity/2 -> signal_valid_out/1
  signal_in/1 -> can_signal_process_wio_validity/1
  msg_valid/1 -> can_signal_process_wio_validity/2
  defval/1 -> can_signal_process_wio_validity/4
  invalid/1 -> can_signal_process_wio_validity/5
  setvalid/1 -> can_signal_process_wio_validity/6
  ovrdflg/1 -> can_signal_process_wio_validity/7
  ovrdval/1 -> can_signal_process_wio_validity/8
  srvcflg/1 -> can_signal_process_wio_validity/9
  srvcval/1 -> can_signal_process_wio_validity/10
  Active_fault_flg/1 -> can_signal_process_wio_validity/3

== system inp/INP/ABS_signals/TCSFault ==
  signal_in : Inport
  msg_valid : Inport  Port=2
  Active_fault_flg : Inport  Port=3
  can_signal_process_wio_validity : inp/can_signal_process_wio_validity
  defval : Constant  Value=KINP_TCSFault_flg_defval
  invalid : Constant  Value=KINP_TCSFault_flg_invalid
  ovrdflg : Constant  Value=KINP_TCSFault_flg_ovrdflg
  ovrdval : Constant  Value=KINP_TCSFault_flg_ovrdval
  setvalid : Constant  Value=KINP_TCSFault_flg_setvalid
  srvcflg : Constant  Value=KINP_TCSFault_flg_srvcflg
  srvcval : Constant  Value=KINP_TCSFault_flg_srvcval
  signal_out : Outport
  signal_valid_out : Outport  Port=2
  wires:
  Active_fault_flg/1 -> can_signal_process_wio_validity/3
  srvcval/1 -> can_signal_process_wio_validity/10
  srvcflg/1 -> can_signal_process_wio_validity/9
  ovrdval/1 -> can_signal_process_wio_validity/8
  ovrdflg/1 -> can_signal_process_wio_validity/7
  setvalid/1 -> can_signal_process_wio_validity/6
  invalid/1 -> can_signal_process_wio_validity/5
  defval/1 -> can_signal_process_wio_validity/4
  msg_valid/1 -> can_signal_process_wio_validity/2
  signal_in/1 -> can_signal_process_wio_validity/1
  can_signal_process_wio_validity/2 -> signal_valid_out/1
  can_signal_process_wio_validity/1 -> signal_out/1

== system inp/INP/ABS_signals/VehicleSpeed ==
  signal_in : Inport
  signal_valid_in : Inport  Port=2
  msg_valid : Inport  Port=3
  Active_fault_flg : Inport  Port=4
  can_signal_process_with_validity : inp/can_signal_process_with_validity
  conv_gain_offset : Subsystem
  defval : Constant  Value=KINP_VehSpd_kph_defval
  invalid : Constant  Value=KINP_VehSpd_kph_invalid
  min_max_limit : Subsystem
  ovrdflg : Constant  Value=KINP_VehSpd_kph_ovrdflg
  ovrdval : Constant  Value=KINP_VehSpd_kph_ovrdval
  setvalid : Constant  Value=KINP_VehSpd_kph_setvalid
  srvcflg : Constant  Value=KINP_VehSpd_kph_srvcflg
  srvcval : Constant  Value=KINP_VehSpd_kph_srvcval
  signal_out : Outport
  signal_valid_out : Outport  Port=2
  raw_signal_valid_out : Outport  Port=3
  wires:
  Active_fault_flg/1 -> can_signal_process_with_validity/4
  srvcval/1 -> can_signal_process_with_validity/12
  srvcflg/1 -> can_signal_process_with_validity/11
  ovrdval/1 -> can_signal_process_with_validity/10
  ovrdflg/1 -> can_signal_process_with_validity/9
  setvalid/1 -> can_signal_process_with_validity/8
  min_max_limit/1(out) -> signal_out/1
  conv_gain_offset/1(out) -> can_signal_process_with_validity/1
  invalid/1 -> can_signal_process_with_validity/7
  defval/1 -> can_signal_process_with_validity/6
  msg_valid/1 -> can_signal_process_with_validity/3, can_signal_process_with_validity/5
  signal_valid_in/1 -> can_signal_process_with_validity/2
  signal_in/1 -> conv_gain_offset/1(in)
  can_signal_process_with_validity/2 -> signal_valid_out/1
  can_signal_process_with_validity/1 -> min_max_limit/1(in)
  can_signal_process_with_validity/3 -> raw_signal_valid_out/1

== system inp/INP/ABS_signals/YawRate ==
  signal_in : Inport
  signal_valid_in : Inport  Port=2
  msg_valid : Inport  Port=3
  Active_fault_flg : Inport  Port=4
  can_signal_process_with_validity : inp/can_signal_process_with_validity
  conv_gain_offset : Subsystem
  defval : Constant  Value=KINP_YawRate_degps_defval
  invalid : Constant  Value=KINP_YawRate_degps_invalid
  min_max_limit : Subsystem
  ovrdflg : Constant  Value=KINP_YawRate_degps_ovrdflg
  ovrdval : Constant  Value=KINP_YawRate_degps_ovrdval
  setvalid : Constant  Value=KINP_YawRate_degps_setvalid
  srvcflg : Constant  Value=KINP_YawRate_degps_srvcflg
  srvcval : Constant  Value=KINP_YawRate_degps_srvcval
  signal_out : Outport
  signal_valid_out : Outport  Port=2
  raw_signal_valid_out : Outport  Port=3
  wires:
  Active_fault_flg/1 -> can_signal_process_with_validity/4
  srvcval/1 -> can_signal_process_with_validity/12
  srvcflg/1 -> can_signal_process_with_validity/11
  ovrdval/1 -> can_signal_process_with_validity/10
  ovrdflg/1 -> can_signal_process_with_validity/9
  setvalid/1 -> can_signal_process_with_validity/8
  min_max_limit/1(out) -> signal_out/1
  conv_gain_offset/1(out) -> can_signal_process_with_validity/1
  invalid/1 -> can_signal_process_with_validity/7
  defval/1 -> can_signal_process_with_validity/6
  msg_valid/1 -> can_signal_process_with_validity/3, can_signal_process_with_validity/5
  signal_valid_in/1 -> can_signal_process_with_validity/2
  signal_in/1 -> conv_gain_offset/1(in)
  can_signal_process_with_validity/2 -> signal_valid_out/1
  can_signal_process_with_validity/1 -> min_max_limit/1(in)
  can_signal_process_with_validity/3 -> raw_signal_valid_out/1

== system inp/INP/AC_signals/OutsideTemp ==
  signal_in : Inport
  signal_valid_in : Inport  Port=2
  msg_valid : Inport  Port=3
  Active_fault_flg : Inport  Port=4
  can_signal_process_with_validity : inp/can_signal_process_with_validity
  conv_gain_offset : Subsystem
  defval : Constant  Value=KINP_OutsideTemp_degC_defval
  invalid : Constant  Value=KINP_OutsideTemp_degC_invalid
  min_max_limit : Subsystem
  ovrdflg : Constant  Value=KINP_OutsideTemp_degC_ovrdflg
  ovrdval : Constant  Value=KINP_OutsideTemp_degC_ovrdval
  setvalid : Constant  Value=KINP_OutsideTemp_degC_setvalid
  srvcflg : Constant  Value=KINP_OutsideTemp_degC_srvcflg
  srvcval : Constant  Value=KINP_OutsideTemp_degC_srvcval
  signal_out : Outport
  signal_valid_out : Outport  Port=2
  raw_signal_valid_out : Outport  Port=3
  wires:
  can_signal_process_with_validity/1 -> min_max_limit/1(in)
  can_signal_process_with_validity/2 -> signal_valid_out/1
  signal_in/1 -> conv_gain_offset/1(in)
  signal_valid_in/1 -> can_signal_process_with_validity/2
  msg_valid/1 -> can_signal_process_with_validity/3, can_signal_process_with_validity/5
  defval/1 -> can_signal_process_with_validity/6
  invalid/1 -> can_signal_process_with_validity/7
  conv_gain_offset/1(out) -> can_signal_process_with_validity/1
  min_max_limit/1(out) -> signal_out/1
  setvalid/1 -> can_signal_process_with_validity/8
  ovrdflg/1 -> can_signal_process_with_validity/9
  ovrdval/1 -> can_signal_process_with_validity/10
  srvcflg/1 -> can_signal_process_with_validity/11
  srvcval/1 -> can_signal_process_with_validity/12
  Active_fault_flg/1 -> can_signal_process_with_validity/4
  can_signal_process_with_validity/3 -> raw_signal_valid_out/1

== system inp/INP/BCM_signals/AnyDoorOpen ==
  signal_in : Inport
  msg_valid : Inport  Port=2
  Active_fault_flg : Inport  Port=3
  can_signal_process_wio_validity : inp/can_signal_process_wio_validity
  defval : Constant  Value=KINP_AnyDoorOpen_flg_defval
  invalid : Constant  Value=KINP_AnyDoorOpen_flg_invalid
  ovrdflg : Constant  Value=KINP_AnyDoorOpen_flg_ovrdflg
  ovrdval : Constant  Value=KINP_AnyDoorOpen_flg_ovrdval
  setvalid : Constant  Value=KINP_AnyDoorOpen_flg_setvalid
  srvcflg : Constant  Value=KINP_AnyDoorOpen_flg_srvcflg
  srvcval : Constant  Value=KINP_AnyDoorOpen_flg_srvcval
  signal_out : Outport
  signal_valid_out : Outport  Port=2
  wires:
  can_signal_process_wio_validity/1 -> signal_out/1
  can_signal_process_wio_validity/2 -> signal_valid_out/1
  signal_in/1 -> can_signal_process_wio_validity/1
  msg_valid/1 -> can_signal_process_wio_validity/2
  defval/1 -> can_signal_process_wio_validity/4
  invalid/1 -> can_signal_process_wio_validity/5
  setvalid/1 -> can_signal_process_wio_validity/6
  ovrdflg/1 -> can_signal_process_wio_validity/7
  ovrdval/1 -> can_signal_process_wio_validity/8
  srvcflg/1 -> can_signal_process_wio_validity/9
  srvcval/1 -> can_signal_process_wio_validity/10
  Active_fault_flg/1 -> can_signal_process_wio_validity/3

== system inp/INP/BCM_signals/DriverDoorOpen ==
  signal_in : Inport
  msg_valid : Inport  Port=2
  Active_fault_flg : Inport  Port=3
  can_signal_process_wio_validity : inp/can_signal_process_wio_validity
  defval : Constant  Value=KINP_DriverDoorOpen_flg_defval
  invalid : Constant  Value=KINP_DriverDoorOpen_flg_invalid
  ovrdflg : Constant  Value=KINP_DriverDoorOpen_flg_ovrdflg
  ovrdval : Constant  Value=KINP_DriverDoorOpen_flg_ovrdval
  setvalid : Constant  Value=KINP_DriverDoorOpen_flg_setvalid
  srvcflg : Constant  Value=KINP_DriverDoorOpen_flg_srvcflg
  srvcval : Constant  Value=KINP_DriverDoorOpen_flg_srvcval
  signal_out : Outport
  signal_valid_out : Outport  Port=2
  wires:
  Active_fault_flg/1 -> can_signal_process_wio_validity/3
  srvcval/1 -> can_signal_process_wio_validity/10
  srvcflg/1 -> can_signal_process_wio_validity/9
  ovrdval/1 -> can_signal_process_wio_validity/8
  ovrdflg/1 -> can_signal_process_wio_validity/7
  setvalid/1 -> can_signal_process_wio_validity/6
  invalid/1 -> can_signal_process_wio_validity/5
  defval/1 -> can_signal_process_wio_validity/4
  msg_valid/1 -> can_signal_process_wio_validity/2
  signal_in/1 -> can_signal_process_wio_validity/1
  can_signal_process_wio_validity/2 -> signal_valid_out/1
  can_signal_process_wio_validity/1 -> signal_out/1

== system inp/INP/BCM_signals/HazardLightSw ==
  signal_in : Inport
  msg_valid : Inport  Port=2
  Active_fault_flg : Inport  Port=3
  can_signal_process_wio_validity : inp/can_signal_process_wio_validity
  defval : Constant  Value=KINP_HazardLightSw_flg_defval
  invalid : Constant  Value=KINP_HazardLightSw_flg_invalid
  ovrdflg : Constant  Value=KINP_HazardLightSw_flg_ovrdflg
  ovrdval : Constant  Value=KINP_HazardLightSw_flg_ovrdval
  setvalid : Constant  Value=KINP_HazardLightSw_flg_setvalid
  srvcflg : Constant  Value=KINP_HazardLightSw_flg_srvcflg
  srvcval : Constant  Value=KINP_HazardLightSw_flg_srvcval
  signal_out : Outport
  signal_valid_out : Outport  Port=2
  wires:
  Active_fault_flg/1 -> can_signal_process_wio_validity/3
  srvcval/1 -> can_signal_process_wio_validity/10
  srvcflg/1 -> can_signal_process_wio_validity/9
  ovrdval/1 -> can_signal_process_wio_validity/8
  ovrdflg/1 -> can_signal_process_wio_validity/7
  setvalid/1 -> can_signal_process_wio_validity/6
  invalid/1 -> can_signal_process_wio_validity/5
  defval/1 -> can_signal_process_wio_validity/4
  msg_valid/1 -> can_signal_process_wio_validity/2
  signal_in/1 -> can_signal_process_wio_validity/1
  can_signal_process_wio_validity/2 -> signal_valid_out/1
  can_signal_process_wio_validity/1 -> signal_out/1
